simulink model "fx12"  (R2016b) (continued)
== system fx12 == (continued)
      |     mask_enables{ismember(mask_names, 'sample_period')} = 'off';
      | else
      |     mask_enables{ismember(mask_names, 'sample_period')} = 'on';
      | end
      | set_param(gcb, 'MaskEnables', mask_enables);
  dir_x0 : Subsystem  mask{n_chans=512}
      mask prompts: n_chans "Number of Channels"
  dir_x1 : Subsystem  mask{n_chans=512}
      mask prompts: n_chans "Number of Channels"
  edge_detect : Subsystem  AncestorBlock=casper_library_misc/edge_detect  mask(edge_detect){edge=Rising, polarity=Active High, x_in=[0 0.08 0.08 0.16 0.16 0.24 0.24 0.32 0.32 0.4], y_in=[-1 -1  1  1  1  1  1  1  1  1], x_out=[0.6 0.68 0.68 0.76 0.76 0.84 0.84 0.92 0.92 1], y_out=[-1 -1  1  1 -1 -1 -1 -1 -1 -1]}
      mask prompts: edge "Edge Type"; polarity "Output Polarity"; x_in "Input X Positions"; y_in "Input Y Positions"; x_out "Output X Positions"; y_out "Output Y Positions"
      mask help: Output is active for one-cycle after each edge of the specified type (i.e. rising, falling or both).
      mask init:
      | edge_detect_init(gcb,...
      |     'edge',edge,...
      |     'polarity',polarity,...
      |     'x_in',x_in,...
      |     'x_out',x_out,...
      |     'y_in',y_in,...
      |     'y_out',y_out);
      | 
      | x_in_num=str2num(getfield(getfield(get_param(gcb,'UserData'),'parameters'),'x_in'));
      | x_out_num=str2num(getfield(getfield(get_param(gcb,'UserData'),'parameters'),'x_out'));
      | y_in_num=str2num(getfield(getfield(get_param(gcb,'UserData'),'parameters'),'y_in'));
      | y_out_num=str2num(getfield(getfield(get_param(gcb,'UserData'),'parameters'),'y_out'));
      settings: AttributesFormatString=|
      | rising edges
      | active high
      mask Help: Output is active for one-cycle after each edge of the specified type (i.e. rising, falling, or both).  Latency is zero.
      mask TypeOptions edge: Rising | Falling | Both
      mask TypeOptions polarity: Active High | Active Low
      mask Display:
      | plot(x_in_num,0.5+y_in_num*0.2);
      | plot(x_out_num,0.5+y_out_num*0.2);
  edge_detect1 : Subsystem  AncestorBlock=casper_library_misc/edge_detect  mask(edge_detect){edge=Rising, polarity=Active High, x_in=[0 0.08 0.08 0.16 0.16 0.24 0.24 0.32 0.32 0.4], y_in=[-1 -1  1  1  1  1  1  1  1  1], x_out=[0.6 0.68 0.68 0.76 0.76 0.84 0.84 0.92 0.92 1], y_out=[-1 -1  1  1 -1 -1 -1 -1 -1 -1]}
      mask prompts: edge "Edge Type"; polarity "Output Polarity"; x_in "Input X Positions"; y_in "Input Y Positions"; x_out "Output X Positions"; y_out "Output Y Positions"
      mask help: Output is active for one-cycle after each edge of the specified type (i.e. rising, falling or both).
      mask init: same as fx12/edge_detect
      settings: AttributesFormatString=|
      | rising edges
      | active high
      mask Help: Output is active for one-cycle after each edge of the specified type (i.e. rising, falling, or both).  Latency is zero.
      mask TypeOptions edge: Rising | Falling | Both
      mask TypeOptions polarity: Active High | Active Low
      mask Display:
      | plot(x_in_num,0.5+y_in_num*0.2);
      | plot(x_out_num,0.5+y_out_num*0.2);
  edge_detect2 : Subsystem  AncestorBlock=casper_library_misc/edge_detect  mask(edge_detect){edge=Rising, polarity=Active High, x_in=[0 0.08 0.08 0.16 0.16 0.24 0.24 0.32 0.32 0.4], y_in=[-1 -1  1  1  1  1  1  1  1  1], x_out=[0.6 0.68 0.68 0.76 0.76 0.84 0.84 0.92 0.92 1], y_out=[-1 -1  1  1 -1 -1 -1 -1 -1 -1]}
      mask prompts: edge "Edge Type"; polarity "Output Polarity"; x_in "Input X Positions"; y_in "Input Y Positions"; x_out "Output X Positions"; y_out "Output Y Positions"
      mask help: Output is active for one-cycle after each edge of the specified type (i.e. rising, falling or both).
      mask init: same as fx12/edge_detect
      settings: AttributesFormatString=|
      | rising edges
      | active high
      mask Help: Output is active for one-cycle after each edge of the specified type (i.e. rising, falling, or both).  Latency is zero.
      mask TypeOptions edge: Rising | Falling | Both
      mask TypeOptions polarity: Active High | Active Low
      mask Display:
      | plot(x_in_num,0.5+y_in_num*0.2);
      | plot(x_out_num,0.5+y_out_num*0.2);
  edge_detect3 : Subsystem  AncestorBlock=casper_library_misc/edge_detect  mask(edge_detect){edge=Rising, polarity=Active High, x_in=[0 0.08 0.08 0.16 0.16 0.24 0.24 0.32 0.32 0.4], y_in=[-1 -1  1  1  1  1  1  1  1  1], x_out=[0.6 0.68 0.68 0.76 0.76 0.84 0.84 0.92 0.92 1], y_out=[-1 -1  1  1 -1 -1 -1 -1 -1 -1]}
      mask prompts: edge "Edge Type"; polarity "Output Polarity"; x_in "Input X Positions"; y_in "Input Y Positions"; x_out "Output X Positions"; y_out "Output Y Positions"
      mask help: Output is active for one-cycle after each edge of the specified type (i.e. rising, falling or both).
      mask init: same as fx12/edge_detect
      settings: AttributesFormatString=|
      | rising edges
      | active high
      mask Help: Output is active for one-cycle after each edge of the specified type (i.e. rising, falling, or both).  Latency is zero.
      mask TypeOptions edge: Rising | Falling | Both
      mask TypeOptions polarity: Active High | Active Low
      mask Display:
      | plot(x_in_num,0.5+y_in_num*0.2);
      | plot(x_out_num,0.5+y_out_num*0.2);
  fft_shift : Subsystem  Tag=xps:sw_reg  AncestorBlock=xps_library/software_register  mask(swreg){io_dir=From Processor, io_delay=0, sample_period=1, names=reg, bitwidths=32, bin_pts=0, arith_types=0, sim_port=on, show_format=on}
      mask prompts: io_dir "I/O direction"; io_delay "I/O delay"; sample_period "Sample period"; names "Bitfield names [msb...lsb]"; bitwidths "Bitfield widths"; bin_pts "Bitfield binary pts"; arith_types "Bitfield types, ufix=0, fix=1, bool=2"; sim_port "Provide sim input/output?"; show_format "Print format string?"
      mask help: A 32-bit software-accessible register. Can be divided into bitfields of varying widths and types using the fields in the block mask.
      mask init:
      | swreg_init(gcb)
      settings: AttributesFormatString=1input:uf32.0 = 32 bits
      mask TypeOptions io_dir: To Processor | From Processor
      mask Callback io_dir:
      | mask_enables = get_param(gcb, 'MaskEnables');
      | mask_names = get_param(gcb, 'MaskNames');
      | if strcmp(get_param(gcb, 'io_dir'), 'To Processor'),
      |     mask_enables{ismember(mask_names, 'sample_period')} = 'off';
      | else
      |     mask_enables{ismember(mask_names, 'sample_period')} = 'on';
      | end
      | set_param(gcb, 'MaskEnables', mask_enables);
      mask DialogControl ParameterTabVar0 Prompt: Setup
  fft_wideband_real : Subsystem  Tag=casper:fft_wideband_real  AncestorBlock=casper_library_ffts/fft_wideband_real  mask(fft_wideband_real){n_streams=3, FFTSize=11, n_inputs=2, input_bit_width=18, bin_pt_in=17, coeff_bit_width=18, unscramble=on, async=off, add_latency=1, mult_latency=3, bram_latency=3, conv_latency=1, input_latency=0, biplex_direct_latency=0, quantization=Round  (unbiased: Even Values), overflow=Wrap, delays_bit_limit=7, coeffs_bit_limit=7, coeff_sharing=off, coeff_decimation=off, coeff_generation=off, cal_bits=1, n_bits_rotation=25, mult_spec=2, max_fanout=8, bitgrowth=off, max_bits=19, hardcode_shifts=off, shift_schedule=[], dsp48_adders=off}
      mask prompts: n_streams "Number simultaneous streams"; FFTSize "Size of FFT: (2^? pts)"; n_inputs "Number of Simultaneous Inputs: (2^?)"; input_bit_width "Input Bit Width"; bin_pt_in "Input binary point"; coeff_bit_width "Coefficient Bit Width"; unscramble "Unscramble output (ie, put channels in canonical order)"; async "Asynchronous operation"; add_latency "Add Latency"; mult_latency "Mult Latency"; bram_latency "BRAM Latency"; conv_latency "Convert Latency"; input_latency "Input Latency"; biplex_direct_latency "Latency between biplexes and fft_direct"; quantization "Quantization Behavior"; overflow "Overflow Behavior"; delays_bit_limit "Number bits above which to store stage's delays in BRAM (2^? bits)"; coeffs_bit_limit "Number bits above which to store stage's coefficients in BRAM (2^? bits)"; coeff_sharing "BRAM sharing in coeff storage"; coeff_decimation "Store a fraction of coeff factors where useful"; coeff_generation "Generate coeffs with multipliers where useful"; cal_bits "Number calibration locations when generating coeffs (2^?)"; n_bits_rotation "Feedback rotation vector resolution"; mult_spec "Multiplier impl. (0=core, 1=embedded, 2=behavioural) (left=1st stage, or just one value)"; max_fanout "Maximum fanout"; bitgrowth "Bitgrowth instead of shifting"; max_bits "Max bits to grow to"; hardcode_shifts "Hardcode shift schedule"; shift_schedule "Shift schedule"; dsp48_adders "DSP48 adders in butterfly"
      mask help: A real-sampled wideband FFT.
      mask init:
      | fft_wideband_real_init(gcb, ...
      |     'n_streams', n_streams, ...
      |     'FFTSize', FFTSize, ...
      |     'n_inputs', n_inputs, ...
      |     'input_bit_width', input_bit_width, ...
      |     'bin_pt_in', bin_pt_in, ...
      |     'coeff_bit_width', coeff_bit_width, ...
      |     'unscramble', unscramble, ...
      |     'async', async, ...
      |     'add_latency', add_latency, ...
      |     'mult_latency', mult_latency, ...
      |     'bram_latency', bram_latency, ...
      |     'conv_latency', conv_latency, ...
      |     'input_latency', input_latency, ...
      |     'biplex_direct_latency', biplex_direct_latency, ...
      |     'quantization', quantization, ...
      |     'overflow', overflow, ...
      |     'delays_bit_limit', delays_bit_limit, ...
      |     'coeffs_bit_limit', coeffs_bit_limit, ...
      |     'coeff_sharing', coeff_sharing, ...
      |     'coeff_decimation', coeff_decimation, ...
      |     'coeff_generation', coeff_generation, ...
      |     'cal_bits', cal_bits, ...
      |     'n_bits_rotation', n_bits_rotation, ...
      |     'max_fanout', max_fanout, ...   
      |     'mult_spec', mult_spec, ...
      |     'bitgrowth', bitgrowth, ...
      |     'max_bits', max_bits, ...
      |     'hardcode_shifts', hardcode_shifts, ...
      |     'shift_schedule', shift_schedule, ...
      |     'dsp48_adders', dsp48_adders);
      settings: AttributesFormatString=|
      | 11 stages
      | (18,18)
      | Round  (unbiased: Even Values)
      | Wrap
      mask Help: eval('xlWeb(''http://casper.berkeley.edu/wiki/Fft_wideband_real'')')
      mask TypeOptions quantization: Truncate | Round  (unbiased: +/- Inf) | Round  (unbiased: Even Values)
      mask TypeOptions overflow: Wrap | Saturate | Error
      mask Callback hardcode_shifts:
      | hardcode_shifts = get_param(gcb, 'hardcode_shifts');
      | set_param_state(gcb, 'shift_schedule', hardcode_shifts);
      mask Callback dsp48_adders: fft_callback_dsp48_adders(gcb);
  i2c_ant1 : xps_library/i2c  Tag=xps:i2c  sda_gpio='rpi_header'  sda_gpio_index=6  scl_gpio='rpi_header'  scl_gpio_index=7
  i2c_ant2 : xps_library/i2c  Tag=xps:i2c  sda_gpio='rpi_header'  sda_gpio_index=30  scl_gpio='rpi_header'  scl_gpio_index=31
  i2c_ant3 : xps_library/i2c  Tag=xps:i2c  sda_gpio='rpi_header'  sda_gpio_index=35  scl_gpio='rpi_header'  scl_gpio_index=36
  led1_mrst : xps_library/gpio  Tag=xps:gpio  io_group=ROACH:led  io_group_custom=0  io_dir=out  arith_type=Boolean  bitwidth=1  bin_pt=0  bit_index=1  sample_period=1  use_single_ended=off  use_ddr=off  reg_iob=on  reg_clk_phase=0  termination=None
  led2_sync : xps_library/gpio  Tag=xps:gpio  io_group=ROACH:led  io_group_custom=0  io_dir=out  arith_type=Boolean  bitwidth=1  bin_pt=0  bit_index=2  sample_period=1  use_single_ended=off  use_ddr=off  reg_iob=on  reg_clk_phase=0  termination=None
  led3_new_acc : xps_library/gpio  Tag=xps:gpio  io_group=ROACH:led  io_group_custom=0  io_dir=out  arith_type=Unsigned  bitwidth=1  bin_pt=0  bit_index=3  sample_period=1  use_single_ended=off  use_ddr=off  reg_iob=on  reg_clk_phase=0  termination=None
  negedge_delay1 : casper_library_misc/negedge_delay  pulse_len=2^24
  pfb_fir_generic : Subsystem  Tag=casper:pfb_fir_generic  AncestorBlock=casper_library_pfbs/pfb_fir_generic  mask(pfb_fir_generic){n_streams=3, PFBSize=11, TotalTaps=4, WindowType=hamming, n_inputs=2, BitWidthIn=16, BitWidthOut=18, CoeffBitWidth=18, complex=off, async=off, add_latency=2, mult_latency=3, bram_latency=1, fan_latency=1, conv_latency=1, quantization=Round  (unbiased: Even Values), fwidth=1, coeffs_bram_optimization=Area, delays_bram_optimization=Area}
      mask prompts: n_streams "Number input streams?"; PFBSize "Size of PFB: (2^? pnts)"; TotalTaps "Total Number of Taps:"; WindowType "Windowing Function:"; n_inputs "Number of Simultaneous Inputs: (2^?)"; BitWidthIn "Input Bitwidth:"; BitWidthOut "Output Bitwidth:"; CoeffBitWidth "Coefficient Bitwidth:"; complex "Complex data inputs"; async "Asynchronous operation"; add_latency "Add Latency"; mult_latency "Mult Latency"; bram_latency "BRAM Latency"; fan_latency "Fanout Latency"; conv_latency "Convert latency"; quantization "Quantization Behavior"; fwidth "Bin Width Scaling (normal=1)"; coeffs_bram_optimization "Coefficient BRAMs optimization"; delays_bram_optimization "Delay BRAMs optimization"
      mask init:
      | pfb_fir_generic_init(gcb, ...
      |     'n_streams', n_streams, ...
      |     'PFBSize', PFBSize, ...
      |     'TotalTaps', TotalTaps, ...
      |     'WindowType', WindowType, ...
      |     'n_inputs', n_inputs, ...
      |     'complex', complex, ...
      |     'BitWidthIn', BitWidthIn, ...
      |     'BitWidthOut', BitWidthOut, ...
      |     'CoeffBitWidth', CoeffBitWidth, ...
      |     'async', async, ...
      |     'add_latency', add_latency, ...
      |     'mult_latency', mult_latency, ...
      |     'bram_latency', bram_latency, ...
      |     'fan_latency', fan_latency, ...
      |     'conv_latency', conv_latency, ...
      |     'quantization', quantization, ...
      |     'fwidth', fwidth, ...
      |     'coeffs_bram_optimization', coeffs_bram_optimization, ...
      |     'delays_bram_optimization', delays_bram_optimization);
      mask Callback TotalTaps:
      | %num_taps = eval(get_param(gcb, 'TotalTaps'));
      | %old_vec = eval(get_param(gcb, 'mult_spec'));
      | 
      | %vec = 2.*ones(1,num_taps);
      | %old_size = length(old_vec);
      | 
      | %if(old_size <= num_taps),
      | %   new_size = old_size;
      | %else,
      | %    new_size = num_taps;
      | %end
      | 
      | %for n = 1:new_size, vec(n) = old_vec(n); end
      | %set_param(gcb, 'mult_spec', mat2str(vec));
      mask TypeOptions WindowType: bartlett | barthannwin | blackman | blackmanharris | bohamwin | chebwin | flattopwin | gausswin | hamming | hann | kaiser | nuttallwin | parzenwin | rectwin | tukeywin | triang | userwindow
      mask TypeOptions quantization: Truncate | Round  (unbiased: +/- Inf) | Round  (unbiased: Even Values)
      mask Callback fwidth:
      | %num_taps = eval(get_param(gcb, 'TotalTaps'));
      | %old_vec = eval(get_param(gcb, 'mult_spec'));
      | 
      | %vec = 2.*ones(1,num_taps);
      | %old_size = length(old_vec);
      | 
      | %if(old_size <= num_taps),
      | %    new_size = old_size;
      | %else,
      | %    new_size = num_taps;
      | %end
      | 
      | %for n = 1:new_size, vec(n) = old_vec(n); end
      | %set_param(gcb, 'mult_spec', mat2str(vec));
      mask TypeOptions coeffs_bram_optimization: Area | Speed
      mask TypeOptions delays_bram_optimization: Area | Speed
  pps_in : xps_library/gpio  Tag=xps:gpio  io_group=ROACH2:sync_in  io_group_custom=0  io_dir=in  arith_type=Boolean  bitwidth=1  bin_pt=0  bit_index=0  sample_period=1  use_single_ended=off  use_ddr=off  reg_iob=off  reg_clk_phase=0  termination=None
  pulse_ext{,1,3..4} (4 alike) : casper_library_misc/pulse_ext  pulse_len=2^24
  pulse_ext2 : Subsystem  mask{pulse_len=2^24}
      mask prompts: pulse_len "Length of Pulse"
      mask init:
      | bits = ceil(log2(pulse_len+1));
  pulse_ext6 : Subsystem  mask{pulse_len=2^24}
      mask prompts: pulse_len "Length of Pulse"
      mask init:
      | bits = ceil(log2(pulse_len+1));
  quant0 : Subsystem
  quant1 : Subsystem
  quant2 : Subsystem
  snap_adc : Subsystem  Tag=xps:snap_adc  AncestorBlock=xps_library/ADCs/snap_adc  mask(adc_snap){sample_rate=640, snap_inputs=3, adc_resolution=12}
      mask prompts: sample_rate "Sample rate (MHz)"; snap_inputs "Number of inputs per snap"; adc_resolution "Resolution of ADC (number of bit)"
      mask help: Interface to the 3 adc chips on the SNAP board. Please see Help for instructions on parameter settings
      mask init:
      | adc_snap_init(gcb);
      mask Help:
      | This is the CASPER "yellow block" for the ADCs on the SNAP board.
      | Each ADC chip accepts four inputs.
      | 
      | Maximum sample rate f (Mega samples per second)
      | 
      | Number of inputs         3                6                12
      | Resolution 8 bits          f=1000      f=500         f=250
      | Resolution 12 bits        f=640        f=320         f=160
      | 
      | Resolution 12 bits is available when SNAP board is upgraded with HMCAD1520 ADC chips
      mask TypeOptions snap_inputs: 3 | 6 | 12
      mask TypeOptions adc_resolution: 8 | 12
  snapshot_eq : Subsystem  Tag=casper:bitsnap  AncestorBlock=casper_library_scopes/bitfield snapshot  mask(bitsnap){io_names=[quant0 quant1 quant2], io_widths=[16 16 16], io_bps=[0 0 0], io_types=[0 0 0], show_format=on, snap_storage=bram, snap_dram_dimm=2, snap_dram_clock=250, snap_nsamples=10, snap_data_width=64, snap_offset=off, snap_circap=off, snap_value=off, snap_use_dsp48=on, snap_delay=0, extra_names=[c d], extra_widths=[30 2], extra_bps=[0 0], extra_types=[0 0]}
      mask prompts: io_names "Names [msb...lsb]"; io_widths "Bitwidths [msb...lsb]"; io_bps "Binary pts [msb...lsb]"; io_types "Data types [msb...lsb], ufix=0, fix=1, bool=2"; show_format "Print format string?"; snap_storage "Storage medium"; snap_dram_dimm "DRAM dimm"; snap_dram_clock "DRAM clock rate"; snap_nsamples "Number of Samples (2^?)"; snap_data_width "Data width"; snap_offset "Start delay support"; snap_circap "Circular capture support"; snap_value "Extra value capture support"; snap_use_dsp48 "Use DSP48s to implement counters"; snap_delay "Pre-snapshot delay"; extra_names "Names [msb...lsb]"; extra_widths "Widths [msb...lsb]"; extra_bps "Binary pts [msb...lsb]"; extra_types "Data types [msb...lsb], ufix=0, fix=1, bool=2"
      mask help:
      | A wrapped snapshot block. Allows specification of inputs to a snapblock word so the toolflow can automatically set up the scope.
      | See documentation for snapshot for explanation of parameters.
      mask init:
      | bitsnap_init(gcb);
      settings: AttributesFormatString=snap(3):uf16.0,uf16.0,uf16.0 = 48 bits
      mask TypeOptions snap_storage: bram | dram
      mask Callback snap_storage: bitsnap_callback();
      mask TypeOptions snap_dram_dimm: 1 | 2 | 3 | 4
      mask TypeOptions snap_dram_clock: 200 | 250 | 266 | 300 | 333
      mask TypeOptions snap_data_width: 8 | 16 | 32 | 64 | 128
      mask Callback snap_value: bitsnap_callback();
  snapshot_fft2 : Subsystem  Tag=casper:bitsnap  AncestorBlock=casper_library_scopes/bitfield snapshot  mask(bitsnap){io_names=[oddreal oddimag evenreal evenimag], io_widths=[32 32 32 32], io_bps=[0 0 0 0], io_types=[0 0 0 0], show_format=on, snap_storage=bram, snap_dram_dimm=2, snap_dram_clock=250, snap_nsamples=9, snap_data_width=128, snap_offset=off, snap_circap=off, snap_value=off, snap_use_dsp48=on, snap_delay=0, extra_names=[c d], extra_widths=[30 2], extra_bps=[0 0], extra_types=[0 0]}
      mask prompts: io_names "Names [msb...lsb]"; io_widths "Bitwidths [msb...lsb]"; io_bps "Binary pts [msb...lsb]"; io_types "Data types [msb...lsb], ufix=0, fix=1, bool=2"; show_format "Print format string?"; snap_storage "Storage medium"; snap_dram_dimm "DRAM dimm"; snap_dram_clock "DRAM clock rate"; snap_nsamples "Number of Samples (2^?)"; snap_data_width "Data width"; snap_offset "Start delay support"; snap_circap "Circular capture support"; snap_value "Extra value capture support"; snap_use_dsp48 "Use DSP48s to implement counters"; snap_delay "Pre-snapshot delay"; extra_names "Names [msb...lsb]"; extra_widths "Widths [msb...lsb]"; extra_bps "Binary pts [msb...lsb]"; extra_types "Data types [msb...lsb], ufix=0, fix=1, bool=2"
      mask help:
      | A wrapped snapshot block. Allows specification of inputs to a snapblock word so the toolflow can automatically set up the scope.
      | See documentation for snapshot for explanation of parameters.
      mask init:
      | bitsnap_init(gcb);
      settings: AttributesFormatString=snap(4):uf32.0,uf32.0,uf32.0,uf32.0 = 128 bits
      mask TypeOptions snap_storage: bram | dram
      mask Callback snap_storage: bitsnap_callback();
      mask TypeOptions snap_dram_dimm: 1 | 2 | 3 | 4
      mask TypeOptions snap_dram_clock: 200 | 250 | 266 | 300 | 333
      mask TypeOptions snap_data_width: 8 | 16 | 32 | 64 | 128
      mask Callback snap_value: bitsnap_callback();
  status : Subsystem  Tag=xps:sw_reg  AncestorBlock=xps_library/software_register  mask(swreg){io_dir=To Processor, io_delay=0, sample_period=1, names=reg, bitwidths=32, bin_pts=0, arith_types=0, sim_port=on, show_format=on}
      mask prompts: io_dir "I/O direction"; io_delay "I/O delay"; sample_period "Sample period"; names "Bitfield names [msb...lsb]"; bitwidths "Bitfield widths"; bin_pts "Bitfield binary pts"; arith_types "Bitfield types, ufix=0, fix=1, bool=2"; sim_port "Provide sim input/output?"; show_format "Print format string?"
      mask help: A 32-bit software-accessible register. Can be divided into bitfields of varying widths and types using the fields in the block mask.
      mask init:
      | swreg_init(gcb)
      settings: AttributesFormatString=1output:uf32.0 = 32 bits
      mask TypeOptions io_dir: To Processor | From Processor
      mask Callback io_dir:
      | mask_enables = get_param(gcb, 'MaskEnables');
      | mask_names = get_param(gcb, 'MaskNames');
      | if strcmp(get_param(gcb, 'io_dir'), 'To Processor'),
      |     mask_enables{ismember(mask_names, 'sample_period')} = 'off';
      | else
      |     mask_enables{ismember(mask_names, 'sample_period')} = 'on';
      | end
      | set_param(gcb, 'MaskEnables', mask_enables);
  sync_gen : Subsystem  mask(sync_gen_hw){sync_period=184320000}
      mask prompts: sync_period "Sync Period"
      mask help:
      | This circuit implements a scheme for synchronizing a design to within an FPGA clock of an external 1 pulse-per-second signal.
      |
      | * An arm pulse will cause the sync_gen to stop issuing sync pulses.
      | * It will then ignore the next input trigger (1PPS), while sync is flushed through the DSP pipeline.
      | * It will then lock onto the next trigger (1PPS) and issue syncs thereafter every sync_period clocks.
      | * You will need a multiple of (pfb_fir taps*LCM(FFT reorder orders) *fft bins/parallel inputs). See the sync memo for further details.
      mask init:
      | sync_period_bits = ceil(log2(sync_period))
  wires:
  bus_create/1(bus_out) -> Delay/1
  sync_gen/1(mrst) -> [mrst]
  sync_gen/2(sync) -> [timing_sync]
  Logical3/1 -> sync_gen/2(pps)
  [soft_sync] -> Logical3/1
  Constant9/1 -> fft_shift/1(sim_1)
  fft_shift/1(in_reg) -> Slice6/1
  quant0/1(sync_out) -> [sync_rnd]
  quant0/3(dout0) -> [eq0]
  quant0/4(dout1) -> [eq1]
  Constant11/1 -> acc_len/1(sim_1)
  acc_len/1(in_reg) -> acc_ctrl/3(acc_len)
  Concat6/1 -> Delay21/1
  Counter1/1 -> acc_num/1(out_reg)
  acc_ctrl/1(new_acc) -> Delay6/1, Delay7/1
  [mrst] -> acc_ctrl/1(mrst)
  Delay5/1 -> [new_acc]
  [sync_rnd] -> acc_ctrl/2(sync)
  acc_num/1(sim_out) -> Scope2/1
  Delay3/1 -> Counter1/2
  [mrst] -> Counter1/1
  Delay6/1 -> dir_x0/1(new_acc), Delay3/1, Delay5/1
  [eq0] -> Concat6/1
  [eq2] -> Concat6/2
  [eq4] -> Concat6/3
  [eq1] -> Concat1/1
  [eq3] -> Concat1/2
  [eq5] -> Concat1/3
  Concat1/1 -> Delay20/1
  Delay7/1 -> dir_x1/1(new_acc)
  Constant1/1 -> ctrl/1(sim_1)
  ctrl/1(in_reg) -> Slice26/1, Slice31/1, Slice32/1
  [clip_rst] -> clip_detect/1(rst)
  Constant24/1 -> clip_detect/30(In_03)
  Constant25/1 -> clip_detect/31(In_02)
  Constant22/1 -> clip_detect/32(In_01)
  Constant23/1 -> clip_detect/33(in_lsb)
  Constant16/1 -> clip_detect/29(In_04)
  Constant17/1 -> clip_detect/26(In_07)
  Constant18/1 -> clip_detect/27(In_06)
  Constant13/1 -> clip_detect/28(In_05)
  [mrst] -> clip_detect/2(in_msb_nr)
  [timing_sync] -> clip_detect/3(In_30_nr)
  [new_acc] -> clip_detect/4(In_29_nr)
  Constant14/1 -> clip_detect/5(In_28_nr)
  Constant19/1 -> clip_detect/6(In_27_nr)
  Constant2/1 -> clip_detect/7(In_26_nr)
  Constant20/1 -> clip_detect/8(In_25_nr)
  Constant21/1 -> clip_detect/9(In_24_nr)
  Constant6/1 -> clip_detect/14(In_19)
  [eq_clip2] -> pulse_ext/1
  [eq_clip1] -> pulse_ext1/1
  [eq_clip0] -> pulse_ext3/1
  Constant7/1 -> clip_detect/18(In_15)
  Constant8/1 -> clip_detect/19(In_14)
  Constant10/1 -> clip_detect/20(In_13)
  Constant12/1 -> clip_detect/21(In_12)
  clip_detect/1(registered_out) -> status/1(out_reg)
  Slice26/1 -> edge_detect/1(in)
  Slice31/1 -> edge_detect1/1(in)
  Slice32/1 -> edge_detect2/1(in)
  edge_detect1/1(out) -> Delay15/1
  edge_detect/1(out) -> Delay16/1
  edge_detect2/1(out) -> Delay4/1
  [sync_arm] -> sync_gen/1(arm)
  Delay/1 -> [adc0]
  pulse_ext2/1(out) -> Inverter2/1
  [timing_sync] -> pulse_ext2/1(in)
  [mrst] -> negedge_delay1/1
  pulse_ext6/1(out) -> Inverter3/1
  [new_acc] -> pulse_ext6/1(in)
  led1_mrst/1 -> Terminator2/1
  led2_sync/1 -> Terminator12/1
  led3_new_acc/1 -> Terminator13/1
  negedge_delay1/1 -> Inverter1/1
  status/1(sim_out) -> Terminator3/1
  Constant15/1 -> clip_detect/10(In_23)
  Constant3/1 -> clip_detect/11(In_22)
  Constant4/1 -> clip_detect/12(In_21)
  Constant5/1 -> clip_detect/13(In_20)
  quant0/2(clip_out) -> [eq_clip0]
  quant1/1(sync_out) -> Terminator8/1
  quant1/3(dout0) -> [eq2]
  quant1/4(dout1) -> [eq3]
  quant1/2(clip_out) -> [eq_clip1]
  quant2/1(sync_out) -> Terminator7/1
  quant2/3(dout0) -> [eq4]
  quant2/2(clip_out) -> [eq_clip2]
  Delay4/1 -> [soft_sync]
  Delay15/1 -> [sync_arm]
  Delay16/1 -> [clip_rst]
  Inverter1/1 -> led1_mrst/1
  Inverter2/1 -> led2_sync/1
  Inverter3/1 -> led3_new_acc/1
  calc_adc_sum_sq1/1(sum_sq) -> adc_sum_sq0/1(out_reg)
  bus_expand6/1(msb_out4) -> calc_adc_sum_sq1/1(In1)
  bus_expand6/2(out3) -> calc_adc_sum_sq1/2(In2)
  bus_expand6/3(out2) -> calc_adc_sum_sq1/3(In3)
  bus_expand6/4(lsb_out1) -> calc_adc_sum_sq1/4(In4)
  adc_sum_sq0/1(sim_out) -> Terminator/1
  calc_adc_sum_sq2/1(sum_sq) -> adc_sum_sq1/1(out_reg)
  bus_expand7/1(msb_out4) -> calc_adc_sum_sq2/1(In1)
  bus_expand7/2(out3) -> calc_adc_sum_sq2/2(In2)
  bus_expand7/3(out2) -> calc_adc_sum_sq2/3(In3)
  bus_expand7/4(lsb_out1) -> calc_adc_sum_sq2/4(In4)
  adc_sum_sq1/1(sim_out) -> Terminator25/1
  calc_adc_sum_sq3/1(sum_sq) -> adc_sum_sq2/1(out_reg)
  bus_expand8/1(msb_out4) -> calc_adc_sum_sq3/1(In1)
  bus_expand8/2(out3) -> calc_adc_sum_sq3/2(In2)
  bus_expand8/3(out2) -> calc_adc_sum_sq3/3(In3)
  bus_expand8/4(lsb_out1) -> calc_adc_sum_sq3/4(In4)
  adc_sum_sq2/1(sim_out) -> Terminator30/1
  [adc1] -> bus_expand7/1(bus_in)
  [adc2] -> bus_expand8/1(bus_in)
  quant2/4(dout1) -> [eq5]
  [fft_over] -> bus_or/1(In1)
  Slice6/1 -> fft_wideband_real/2(shift)
  fft_wideband_real/8(of) -> [fft_over]
  a0p0_1st_shift/1(sync_out) -> fft_wideband_real/1(sync)
  a0p0_1st_shift/10(dout9) -> fft_wideband_real/11(in20)
  a0p0_1st_shift/6(dout5) -> fft_wideband_real/7(in10)
  a0p0_1st_shift/7(dout6) -> fft_wideband_real/8(in11)
  a0p0_1st_shift/8(dout7) -> fft_wideband_real/9(in12)
  a0p0_1st_shift/9(dout8) -> fft_wideband_real/10(in13)
  a0p0_1st_shift/2(dout1) -> fft_wideband_real/3(in00)
  a0p0_1st_shift/3(dout2) -> fft_wideband_real/4(in01)
  a0p0_1st_shift/5(dout4) -> fft_wideband_real/6(in03)
  a0p0_1st_shift/4(dout3) -> fft_wideband_real/5(in02)
  bus_or/1(Out1) -> pulse_ext4/1
  fft_wideband_real/1(sync_out) -> [fft_sync]
  bus_create4/1(bus_out) -> [fft_0]
  [fft_sync] -> quant0/1(sync)
  [fft_sync] -> quant1/1(sync)
  [fft_sync] -> quant2/1(sync)
  [fft_0] -> quant0/2(din)
  [fft_1] -> quant1/2(din)
  [fft_2] -> quant2/2(din)
  fft_wideband_real/5(out11) -> bus_create5/2(in2)
  fft_wideband_real/4(out10) -> bus_create5/1(in1)
  bus_create5/1(bus_out) -> [fft_1]
  bus_create3/1(bus_out) -> [fft_2]
  snap_adc/1(a1) -> bus_create/1(in1)
  snap_adc/2(a2) -> bus_create/2(in2)
  snap_adc/3(a3) -> bus_create/3(in3)
  snap_adc/4(a4) -> bus_create/4(in4)
  bus_create1/1(bus_out) -> Delay1/1
  Delay1/1 -> [adc1]
  snap_adc/5(b1) -> bus_create1/1(in1)
  snap_adc/6(b2) -> bus_create1/2(in2)
  snap_adc/7(b3) -> bus_create1/3(in3)
  snap_adc/8(b4) -> bus_create1/4(in4)
  bus_create2/1(bus_out) -> Delay2/1
  Delay2/1 -> [adc2]
  snap_adc/9(c1) -> bus_create2/1(in1)
  snap_adc/10(c2) -> bus_create2/2(in2)
  snap_adc/11(c3) -> bus_create2/3(in3)
  snap_adc/12(c4) -> bus_create2/4(in4)
  pps_in/1 -> edge_detect3/1(in)
  edge_detect3/1(out) -> Logical3/2
  [timing_sync] -> pfb_fir_generic/1(sync)
  [adc0] -> bus_expand1/1(bus_in)
  bus_expand1/1(msb_out4) -> pfb_fir_generic/2(pol0_in0)
  bus_expand1/2(out3) -> pfb_fir_generic/3(pol0_in1)
  bus_expand1/3(out2) -> pfb_fir_generic/4(pol0_in2)
  bus_expand1/4(lsb_out1) -> pfb_fir_generic/5(pol0_in3)
  [adc1] -> bus_expand2/1(bus_in)
  bus_expand2/1(msb_out4) -> pfb_fir_generic/6(pol1_in0)
  bus_expand2/2(out3) -> pfb_fir_generic/7(pol1_in1)
  bus_expand2/3(out2) -> pfb_fir_generic/8(pol1_in2)
  bus_expand2/4(lsb_out1) -> pfb_fir_generic/9(pol1_in3)
  [adc2] -> bus_expand3/1(bus_in)
  bus_expand3/1(msb_out4) -> pfb_fir_generic/10(pol2_in0)
  bus_expand3/2(out3) -> pfb_fir_generic/11(pol2_in1)
  bus_expand3/3(out2) -> pfb_fir_generic/12(pol2_in2)
  bus_expand3/4(lsb_out1) -> pfb_fir_generic/13(pol2_in3)
  pfb_fir_generic/1(sync_out) -> a0p0_1st_shift/1(sync_in)
  pfb_fir_generic/2(pol0_out0) -> a0p0_1st_shift/2(din1)
  pfb_fir_generic/3(pol0_out1) -> a0p0_1st_shift/3(din2)
  pfb_fir_generic/4(pol0_out2) -> a0p0_1st_shift/4(din3)
  pfb_fir_generic/5(pol0_out3) -> a0p0_1st_shift/5(din4)
  pfb_fir_generic/6(pol1_out0) -> a0p0_1st_shift/6(din5)
  pfb_fir_generic/7(pol1_out1) -> a0p0_1st_shift/7(din6)
  pfb_fir_generic/8(pol1_out2) -> a0p0_1st_shift/8(din7)
  pfb_fir_generic/9(pol1_out3) -> a0p0_1st_shift/9(din8)
  pfb_fir_generic/10(pol2_out0) -> a0p0_1st_shift/10(din9)
  pfb_fir_generic/11(pol2_out1) -> a0p0_1st_shift/11(din10)
  pfb_fir_generic/12(pol2_out2) -> a0p0_1st_shift/12(din11)
  pfb_fir_generic/13(pol2_out3) -> a0p0_1st_shift/13(din12)
  a0p0_1st_shift/11(dout10) -> fft_wideband_real/12(in21)
  a0p0_1st_shift/12(dout11) -> fft_wideband_real/13(in22)
  a0p0_1st_shift/13(dout12) -> fft_wideband_real/14(in23)
  fft_wideband_real/6(out20) -> bus_create3/1(in1)
  fft_wideband_real/7(out21) -> bus_create3/2(in2)
  fft_wideband_real/2(out00) -> bus_create4/1(in1)
  fft_wideband_real/3(out01) -> bus_create4/2(in2)
  pulse_ext/1 -> clip_detect/15(In_18)
  pulse_ext1/1 -> clip_detect/16(In_17)
  pulse_ext3/1 -> clip_detect/17(In_16)
  pulse_ext4/1 -> clip_detect/22(In_11), clip_detect/23(In_10), clip_detect/24(In_09), clip_detect/25(In_08)
  Constant26/1 -> snapshot_eq/4(we)
  [sync_rnd] -> snapshot_eq/5(trig)
  Concat2/1 -> snapshot_eq/1(in_quant0)
  Concat4/1 -> snapshot_eq/2(in_quant1)
  Concat3/1 -> snapshot_eq/3(in_quant2)
  [eq0] -> Concat2/1
  [eq1] -> Concat2/2
  [eq4] -> Concat3/1
  [eq2] -> Concat4/1
  [eq3] -> Concat4/2
  [eq5] -> Concat3/2
  bus_expand4/1(msb_out3) -> dir_x1/2(a)
  bus_expand4/2(out2) -> dir_x1/3(b)
  bus_expand4/3(lsb_out1) -> dir_x1/4(c)
  Delay20/1 -> bus_expand4/1(bus_in)
  bus_expand5/1(msb_out3) -> dir_x0/2(a)
  bus_expand5/2(out2) -> dir_x0/3(b)
  bus_expand5/3(lsb_out1) -> dir_x0/4(c)
  Delay21/1 -> bus_expand5/1(bus_in)
  [adc0] -> bus_expand6/1(bus_in)
  Constant27/1 -> snapshot_fft2/5(we)
  [sync_rnd] -> snapshot_fft2/6(trig)
  [fft_2] -> bus_expand9/1(bus_in)
  bus_expand9/1(msb_out4) -> BitBasher/1
  bus_expand9/2(out3) -> BitBasher1/1
  bus_expand9/3(out2) -> BitBasher2/1
  bus_expand9/4(lsb_out1) -> BitBasher3/1
  BitBasher/1 -> snapshot_fft2/1(in_oddreal)
  BitBasher1/1 -> snapshot_fft2/2(in_oddimag)
  BitBasher2/1 -> snapshot_fft2/3(in_evenreal)
  BitBasher3/1 -> snapshot_fft2/4(in_evenimag)
